simulink model "IEEE_34_node_2024a"  (R2024a)
settings: Solver=FixedStepDiscrete  StartTime=0.0  StopTime=0.001  RelTol=1e-3
InitFcn:
  | power_34NodeTestFeeder_init;
  | power_34NodeTestFeeder_loads_init;
  | %=============================
  | Frequency = 60;
  | 
  | Vnom_ph_ph = 24900;
  | Vnom = Vnom_ph_ph/sqrt(3);
  | 
  | Vnom1_ph_ph = 4160;
  | Vnom1 = Vnom1_ph_ph/sqrt(3);
  | 
  | Apparent_power_gen1 = 2500e3;
  | Degrees_ph_ph_gen1 = 0;
values from neighbouring files (symbols this model uses):
  C_300 = B_300/mi2km*ms2F    [power_34NodeTestFeeder_init.m]
  C_301 = B_301/mi2km*ms2F    [power_34NodeTestFeeder_init.m]
  C_302 = B_302/mi2km*ms2F    [power_34NodeTestFeeder_init.m]
  C_303 = B_303/mi2km*ms2F    [power_34NodeTestFeeder_init.m]
  C_304 = B_304/mi2km*ms2F    [power_34NodeTestFeeder_init.m]
  ft2km = 0.0003048    [power_34NodeTestFeeder_init.m]
  L_300 = X_300/mi2km/2/pi/60    [power_34NodeTestFeeder_init.m]
  L_301 = X_301/mi2km/2/pi/60    [power_34NodeTestFeeder_init.m]
  L_302 = X_302/mi2km/2/pi/60    [power_34NodeTestFeeder_init.m]
  L_303 = X_303/mi2km/2/pi/60    [power_34NodeTestFeeder_init.m]
  L_304 = X_304/mi2km/2/pi/60    [power_34NodeTestFeeder_init.m]
  Pa_802_806 = [1e-6 30 25]*1e3    [power_34NodeTestFeeder_loads_init.m]
  Pa_808_810 = 16e3    (= 16000)    [power_34NodeTestFeeder_loads_init.m]
  Pa_816_824 = [1e-6 5 1e-6]*1e3    [power_34NodeTestFeeder_loads_init.m]
  Pa_818_820 = 34e3    (= 34000)    [power_34NodeTestFeeder_loads_init.m]
  Pa_820_822 = 135e3    (= 135000)    [power_34NodeTestFeeder_loads_init.m]
  Pa_824_826 = 40e3    (= 40000)    [power_34NodeTestFeeder_loads_init.m]
  Pa_824_828 = [1e-6 1e-6 4]*1e3    [power_34NodeTestFeeder_loads_init.m]
  Pa_828_830 = [7 1e-6 1e-6]*1e3    [power_34NodeTestFeeder_loads_init.m]
  Pa_832_858 = [7 2 6]*1e3    [power_34NodeTestFeeder_loads_init.m]
  Pa_834_860 = [16 20 110]*1e3    [power_34NodeTestFeeder_loads_init.m]
  Pa_836_840 = [18 22 1e-6]*1e3    [power_34NodeTestFeeder_loads_init.m]
  Pa_842_844 = [9 1e-6 1e-6]*1e3    [power_34NodeTestFeeder_loads_init.m]
  Pa_844_846 = [1e-6 25 20]*1e3    [power_34NodeTestFeeder_loads_init.m]
  Pa_846_848 = [1e-6 23 1e-6]*1e3    [power_34NodeTestFeeder_loads_init.m]
  Pa_854_856 = 4e3    (= 4000)    [power_34NodeTestFeeder_loads_init.m]
  Pa_858_834 = [4 15 13]*1e3    [power_34NodeTestFeeder_loads_init.m]
  Pa_858_864 = 2e3    (= 2000)    [power_34NodeTestFeeder_loads_init.m]
  Pa_860_836 = [30 10 42]*1e3    [power_34NodeTestFeeder_loads_init.m]
  Pa_862_838 = 28e3    (= 28000)    [power_34NodeTestFeeder_loads_init.m]
  Pp_802_806 = [1e-6 15 14]*1e3    [power_34NodeTestFeeder_loads_init.m]
  Pp_808_810 = 8e3    (= 8000)    [power_34NodeTestFeeder_loads_init.m]
  Pp_816_824 = [1e-6 2 1e-6]*1e3    [power_34NodeTestFeeder_loads_init.m]
  Pp_818_820 = 17e3    (= 17000)    [power_34NodeTestFeeder_loads_init.m]
  Pp_820_822 = 70e3    (= 70000)    [power_34NodeTestFeeder_loads_init.m]
  Pp_824_826 = 20e3    (= 20000)    [power_34NodeTestFeeder_loads_init.m]
  Pp_824_828 = [1e-6 1e-6 2]*1e3    [power_34NodeTestFeeder_loads_init.m]
  Pp_828_830 = [3 1e-6 1e-6]*1e3    [power_34NodeTestFeeder_loads_init.m]
  Pp_832_858 = [3 1 3]*1e3    [power_34NodeTestFeeder_loads_init.m]
  Pp_834_860 = [8 10 55]*1e3    [power_34NodeTestFeeder_loads_init.m]
  Pp_836_840 = [9 11 1e-6]*1e3    [power_34NodeTestFeeder_loads_init.m]
  Pp_842_844 = [5 1e-6 1e-6]*1e3    [power_34NodeTestFeeder_loads_init.m]
  Pp_844_846 = [1e-6 12 11]*1e3    [power_34NodeTestFeeder_loads_init.m]
  Pp_846_848 = [1e-6 11 1e-6]*1e3    [power_34NodeTestFeeder_loads_init.m]
  Pp_854_856 = 2e3    (= 2000)    [power_34NodeTestFeeder_loads_init.m]
  Pp_858_834 = [2 8 7]*1e3    [power_34NodeTestFeeder_loads_init.m]
  Pp_858_864 = 1e3    (= 1000)    [power_34NodeTestFeeder_loads_init.m]
  Pp_860_836 = [15 6 22]*1e3    [power_34NodeTestFeeder_loads_init.m]
  Pp_862_838 = 14e3    (= 14000)    [power_34NodeTestFeeder_loads_init.m]
  R_300 = R_300/mi2km    [power_34NodeTestFeeder_init.m]
  R_301 = R_301/mi2km    [power_34NodeTestFeeder_init.m]
  R_302 = R_302/mi2km    [power_34NodeTestFeeder_init.m]
  R_303 = R_303/mi2km    [power_34NodeTestFeeder_init.m]
  R_304 = R_304/mi2km    [power_34NodeTestFeeder_init.m]
  V_802_806 = [1.047 1.048 1.048]    [power_34NodeTestFeeder_loads_init.m]
  V_808_810 = 1.014    [power_34NodeTestFeeder_loads_init.m]
  V_816_824 = [1.017 1.025 1.02]*sqrt(3)    [power_34NodeTestFeeder_loads_init.m]
  V_818_820 = 1.016    [power_34NodeTestFeeder_loads_init.m]
  V_820_822 = 0.993    [power_34NodeTestFeeder_loads_init.m]
  V_824_826 = 1.008    [power_34NodeTestFeeder_loads_init.m]
  V_824_828 = [1.008 1.016 1.012]    [power_34NodeTestFeeder_loads_init.m]
  V_828_830 = [1.007 1.015 1.011]    [power_34NodeTestFeeder_loads_init.m]
  V_830 = [0.989 0.998 0.994]*sqrt(3)    [power_34NodeTestFeeder_loads_init.m]
  V_832_858 = [1.036 1.035 1.036]*sqrt(3)    [power_34NodeTestFeeder_loads_init.m]
  V_834_860 = [1.031 1.029 1.031]*sqrt(3)    [power_34NodeTestFeeder_loads_init.m]
  V_836_840 = [1.03 1.029 1.031]*sqrt(3)    [power_34NodeTestFeeder_loads_init.m]
  V_840 = [1.03 1.029 1.031]    [power_34NodeTestFeeder_loads_init.m]
  V_842_844 = [1.031 1.029 1.031]    [power_34NodeTestFeeder_loads_init.m]
  V_844 = [1.031 1.029 1.031]    [power_34NodeTestFeeder_loads_init.m]
  V_844_846 = [1.031 1.029 1.031]    [power_34NodeTestFeeder_loads_init.m]
  V_846_848 = [1.031 1.029 1.031]    [power_34NodeTestFeeder_loads_init.m]
  V_848 = [1.031 1.029 1.031]*sqrt(3)    [power_34NodeTestFeeder_loads_init.m]
  V_854_856 = 0.998    [power_34NodeTestFeeder_loads_init.m]
  V_858_834 = [1.034 1.032 1.034]*sqrt(3)    [power_34NodeTestFeeder_loads_init.m]
  V_860 = [1.03 1.029 1.031]    [power_34NodeTestFeeder_loads_init.m]
  V_860_836 = [1.03 1.029 1.031]*sqrt(3)    [power_34NodeTestFeeder_loads_init.m]
  V_862_838 = 1.029    [power_34NodeTestFeeder_loads_init.m]
  V_890 = [0.917 0.924 0.918]*sqrt(3)    [power_34NodeTestFeeder_loads_init.m]
undefined symbols (not defined in the file or in its folder): Angle, D1, Degrees_ph, Delta, Magnitude, ph, ph_gen1, Vnom_ph
libraries: spsLoadFlowBusLib x36, spsDistributedParametersLineLib x32, spsThreePhaseSeriesRLCLoadLib x32, spsThreePhaseVIMeasurementLib x17, spsSeriesRLCLoadLib x14, spsGroundLib x14, spsThreePhaseSourceLib x1, spsThreePhaseTransformerTwoWindingsLib x1, sps_lib x1
(part 1 of 2)
settings Model: Creator=liyifu  LastModifiedBy=GW SmartGrid Lab
settings SolverCC: AlgebraicLoopSolver=TrustRegion
settings DataIOCC: ReturnWorkspaceOutputs=off
settings DebuggingCC: UnconnectedInputMsg=warning  UnconnectedOutputMsg=warning  UnconnectedLineMsg=warning  ArithmeticOperatorsInVariantConditions=warning
settings ModelReferenceCC: EnableRefExpFcnMdlSchedulingChecks=on
settings SFSimCC: SimUseLocalCustomCode=off  CustomCodeUndefinedFunction=UseInterfaceOnly
settings RTWCC: RTWUseLocalCustomCode=off  GPUComputeCapability=3.5

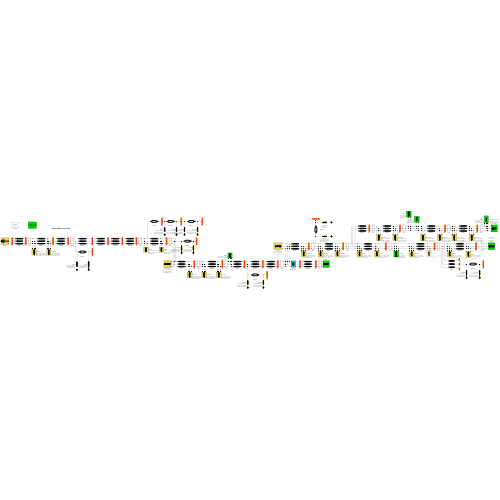
[thumbnail: Simulink's stored preview of the top-level diagram]
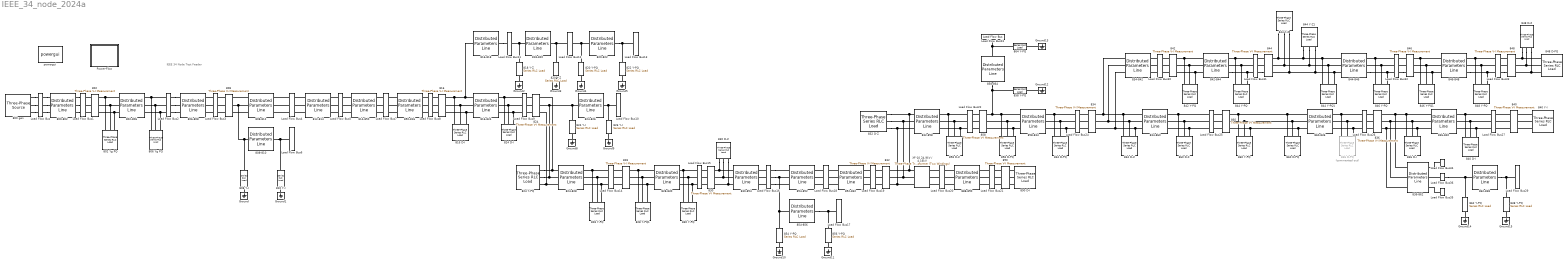
== system IEEE_34_node_2024a ==
  note: IEEE 34 Node Test Feeder
  800 gen : spsThreePhaseSourceLib/Three-Phase Source  InternalConnection=Yg  VoltagePhases=on  Voltage=Vnom_ph-ph  PhaseAngle=Degrees_ph-ph_gen1  Voltage_phases=[1.05 1.05 1.05]*Vnom  PhaseAngles_phases=[0 -120 +120]  Frequency=Frequency  NonIdealSource=off  SpecifyImpedance=on  Resistance=0.8929  Inductance=16.58e-3  ShortCircuitLevel=Apparent_power_gen1  BaseVoltage=Vnom_ph_ph  XRratio=8  BusType=swing  Pref=10e3  Qref=0  Prefabc=[10e3, 10e3, 10e3]  Qrefabc=[0, 0, 0]  Qmin=-inf  Qmax=inf
  800-802 : spsDistributedParametersLineLib/Distributed Parameters Line  Phases=3  Length=2580*ft2km  Frequency=Frequency  Resistance=R_300  Inductance=L_300  Capacitance=C_300  x1=[-3.47e-06 1164.97070083718 7669.53294066039 0;-3.46e-06 1164.9689933214 7669.62711521654 0;-3.45e-06 1164.96728578908 7669.72128966369 0;-3.44e-06 1164.96557824019 7669.81546400184 0;-3.43e-06 1164.96387067475 7669.90963823098 0;-3.42e-06 1164.96216309275 7670.00381235112 0;-3.41e-06 1164.96045549419 7670.09798636224 0;-3.4e-06 1164.95874787908 7670.19216026436 0;-3.39e-06 1164.95704024741 7670.28633405747 0;-3.38e-06 1164.95533259918 7670.38050774156 0;-3.37e-06 1164.9536249344 7670.47468131665 0;-3.36e-06 1164.95191725306 7670.56885478272 0;-3.35e-06 1164.95020955516 7670.66302813977 0;-3.34e-06 1164.94850184071 7670.7572013878 0;-3.33e-06 1164.9467941097 7670.85137452682 0;-3.32e-06 1164.94508636213 7670.94554755682 0;-3.31e-06 1164.94337859801 7671.03972047779 0;-3.3e-06 1164.94167081733 7671.13389328974 0;-3.29e-06 1164.9399630201 7671.22806599267 0;-3.28e-06 1164.93825520631 7671.32223858658 0;-3.27e-06 1164.93654737596 7671.41641107145 0;-3.26e-06 1164.93483952905 7671.5105834473 0;-3.25e-06 1164.93313166559 7671.60475571412 0;-3.24e-06 1164.93142378558 7671.69892787191 0;-3.23e-06 1164.929715889 7671.79309992067 0;-3.22e-06 1164.92800797587 7671.88727186039 0;-3.21e-06 1164.92630004619 7671.98144369108 0;-3.2e-06 1164.92459209995 7672.07561541273 0;-3.19e-06 1164.92288413715 7672.16978702535 0;-3.18e-06 1164.9211761578 7672.26395852893 0;-3.17e-06 1164.91946816189 7672.35812992346 0;-3.16e-06 1164.91776014942 7672.45230120896 0;-3.15e-06 1164.9160521204 7672.54647238541 0;-3.14e-06 1164.91434407482 7672.64064345282 0;-3.13e-06 1164.91263601268 7672.73481441119 0;-3.12e-06 1164.91092793399 7672.8289852605 0;-3.11e-06 1164.90921983875 7672.92315600077 0;-3.1e-06 1164.90751172694 7673.01732663199 0;-3.09e-06 1164.90580359859 7673.11149715416 0;-3.08e-06 1164.90409545367 7673.20566756728 0;-3.07e-06 1164.9023872922 7673.29983787134 0;-3.06e-06 1164.90067911418 7673.39400806635 0;-3.05e-06 1164.89897091959 7673.48817815231 0;-3.04e-06 1164.89726270846 7673.5823481292 0;-3.03e-06 1164.89555448076 7673.67651799704 0;-3.02e-06 1164.89384623651 7673.77068775581 0;-3.01e-06 1164.89213797571 7673.86485740553 0;-3e-06 1164.89042969835 7673.95902694618 0;-2.99e-06 1164.88872140443 7674.05319637777 0;-2.98e-06 1164.88701309396 7674.1473657003 0;-2.97e-06 1164.88530476693 7674.24153491375 0;-2.96e-06 1164.88359642335 7674.33570401814 0;-2.95e-06 1164.88188806321 7674.42987301346 0;-2.94e-06 1164.88017968652 7674.52404189971 0;-2.93e-06 1164.87847129326 7674.61821067688 0;-2.92e-06 1164.87676288346 7674.71237934499 0;-2.91e-06 1164.8750544571 7674.80654790401 0;-2.9e-06 1164.87334601418 7674.90071635397 24835.4217460891;-2.89e-06 1164.87163755471 7674.99488469484 24835.3926245131;-2.88e-06 1164.86992907868 7675.08905292664 24835.3635025841;-2.87e-06 1164.8682205861 7675.18322104935 24835.3343803022;-2.86e-06 1164.86651207696 7675.27738906298 24835.3052576673;-2.85e-06 1164.86480355127 7675.37155696754 24835.2761346794;-2.84e-06 1164.86309500902 7675.465724763 24835.2470113386;-2.83e-06 1164.86138645022 7675.55989244939 24835.2178876448;-2.82e-06 1164.85967787486 7675.65406002668 24835.188763598;-2.81e-06 1164.85796928294 7675.74822749489 24835.1596391983;-2.8e-06 1164.85626067447 7675.842394854 24835.1305144456;-2.79e-06 1164.85455204945 7675.93656210403 24835.10138934;-2.78e-06 1164.85284340787 7676.03072924496 24835.0722638813;-2.77e-06 1164.85113474973 7676.1248962768 24835.0431380698;-2.76e-06 1164.84942607504 7676.21906319955 24835.0140119052;-2.75e-06 1164.84771738379 7676.3132300132 24834.9848853877;-2.74e-06 1164.84600867599 7676.40739671775 24834.9557585173;-2.73e-06 1164.84429995164 7676.5015633132 24834.9266312939;-2.72e-06 1164.84259121073 7676.59572979956 24834.8975037175;-2.71e-06 1164.84088245326 7676.68989617681 24834.8683757881;-2.7e-06 1164.83917367924 7676.78406244496 24834.8392475058;-2.69e-06 1164.83746488866 7676.878228604 24834.8101188706;-2.68e-06 1164.83575608153 7676.97239465394 24834.7809898824;-2.67e-06 ...(+16035 chars)]  x2=[-3.47e-06 1168.50780914208 7624.72535894941 0;-3.46e-06 1168.50605789348 7624.81945720973 0;-3.45e-06 1168.50430662827 7624.91355536169 0;-3.44e-06 1168.50255534646 7625.00765340529 0;-3.43e-06 1168.50080404804 7625.10175134052 0;-3.42e-06 1168.49905273301 7625.19584916737 0;-3.41e-06 1168.49730140137 7625.28994688586 0;-3.4e-06 1168.49555005313 7625.38404449597 0;-3.39e-06 1168.49379868828 7625.47814199771 0;-3.38e-06 1168.49204730682 7625.57223939107 0;-3.37e-06 1168.49029590876 7625.66633667606 0;-3.36e-06 1168.48854449409 7625.76043385267 0;-3.35e-06 1168.48679306282 7625.8545309209 0;-3.34e-06 1168.48504161493 7625.94862788076 0;-3.33e-06 1168.48329015044 7626.04272473222 0;-3.32e-06 1168.48153866934 7626.13682147531 0;-3.31e-06 1168.47978717164 7626.23091811001 0;-3.3e-06 1168.47803565733 7626.32501463633 0;-3.29e-06 1168.47628412641 7626.41911105426 0;-3.28e-06 1168.47453257889 7626.5132073638 0;-3.27e-06 1168.47278101476 7626.60730356495 0;-3.26e-06 1168.47102943402 7626.70139965771 0;-3.25e-06 1168.46927783668 7626.79549564207 0;-3.24e-06 1168.46752622273 7626.88959151805 0;-3.23e-06 1168.46577459217 7626.98368728562 0;-3.22e-06 1168.46402294501 7627.07778294481 0;-3.21e-06 1168.46227128124 7627.17187849559 0;-3.2e-06 1168.46051960086 7627.26597393798 0;-3.19e-06 1168.45876790388 7627.36006927196 0;-3.18e-06 1168.45701619029 7627.45416449754 0;-3.17e-06 1168.45526446009 7627.54825961472 0;-3.16e-06 1168.45351271329 7627.6423546235 0;-3.15e-06 1168.45176094988 7627.73644952387 0;-3.14e-06 1168.45000916987 7627.83054431583 0;-3.13e-06 1168.44825737325 7627.92463899938 0;-3.12e-06 1168.44650556002 7628.01873357452 0;-3.11e-06 1168.44475373019 7628.11282804126 0;-3.1e-06 1168.44300188375 7628.20692239958 0;-3.09e-06 1168.4412500207 7628.30101664948 0;-3.08e-06 1168.43949814105 7628.39511079098 0;-3.07e-06 1168.43774624479 7628.48920482405 0;-3.06e-06 1168.43599433193 7628.58329874871 0;-3.05e-06 1168.43424240246 7628.67739256494 0;-3.04e-06 1168.43249045638 7628.77148627276 0;-3.03e-06 1168.4307384937 7628.86557987216 0;-3.02e-06 1168.42898651441 7628.95967336313 0;-3.01e-06 1168.42723451852 7629.05376674567 0;-3e-06 1168.42548250602 7629.1478600198 0;-2.99e-06 1168.42373047691 7629.24195318549 0;-2.98e-06 1168.4219784312 7629.33604624276 0;-2.97e-06 1168.42022636888 7629.43013919159 0;-2.96e-06 1168.41847428996 7629.524232032 0;-2.95e-06 1168.41672219443 7629.61832476397 0;-2.94e-06 1168.4149700823 7629.71241738751 0;-2.93e-06 1168.41321795355 7629.80650990261 0;-2.92e-06 1168.41146580821 7629.90060230928 0;-2.91e-06 1168.40971364626 7629.9946946075 0;-2.9e-06 1168.4079614677 7630.08878679729 24823.450895793;-2.89e-06 1168.40620927253 7630.18287887864 24823.4219609793;-2.88e-06 1168.40445706076 7630.27697085155 24823.3930258128;-2.87e-06 1168.40270483239 7630.37106271601 24823.3640902935;-2.86e-06 1168.40095258741 7630.46515447203 24823.3351544214;-2.85e-06 1168.39920032582 7630.5592461196 24823.3062181966;-2.84e-06 1168.39744804763 7630.65333765873 24823.2772816189;-2.83e-06 1168.39569575283 7630.74742908941 24823.2483446885;-2.82e-06 1168.39394344143 7630.84152041163 24823.2194074052;-2.81e-06 1168.39219111342 7630.93561162541 24823.1904697692;-2.8e-06 1168.39043876881 7631.02970273073 24823.1615317803;-2.79e-06 1168.38868640759 7631.1237937276 24823.1325934387;-2.78e-06 1168.38693402976 7631.21788461602 24823.1036547443;-2.77e-06 1168.38518163533 7631.31197539597 24823.0747156971;-2.76e-06 1168.3834292243 7631.40606606747 24823.0457762971;-2.75e-06 1168.38167679665 7631.50015663051 24823.0168365443;-2.74e-06 1168.37992435241 7631.59424708509 24822.9878964387;-2.73e-06 1168.37817189156 7631.68833743121 24822.9589559803;-2.72e-06 1168.3764194141 7631.78242766887 24822.9300151692;-2.71e-06 1168.37466692004 7631.87651779805 24822.9010740052;-2.7e-06 1168.37291440937 7631.97060781878 24822.8721324885;-2.69e-06 1168.3711618821 7632.06469773104 24822.8431906189;-2.68e-06 1168.36940933822 7632.15878753482 24822.8142483966 ...(+16047 chars)]  x3=[-3.47e-06 9.92857968224924 -15.9400911726028 0;-3.46e-06 9.9285662285998 -15.9400481932344 0;-3.45e-06 9.92855277480924 -15.9400052136394 0;-3.44e-06 9.92853932087758 -15.9399622338179 0;-3.43e-06 9.92852586680481 -15.9399192537698 0;-3.42e-06 9.92851241259094 -15.9398762734952 0;-3.41e-06 9.92849895823595 -15.939833292994 0;-3.4e-06 9.92848550373987 -15.9397903122663 0;-3.39e-06 9.92847204910267 -15.9397473313121 0;-3.38e-06 9.92845859432437 -15.9397043501313 0;-3.37e-06 9.92844513940497 -15.939661368724 0;-3.36e-06 9.92843168434446 -15.9396183870901 0;-3.35e-06 9.92841822914284 -15.9395754052297 0;-3.34e-06 9.92840477380012 -15.9395324231428 0;-3.33e-06 9.9283913183163 -15.9394894408293 0;-3.32e-06 9.92837786269137 -15.9394464582893 0;-3.31e-06 9.92836440692534 -15.9394034755228 0;-3.3e-06 9.9283509510182 -15.9393604925297 0;-3.29e-06 9.92833749496996 -15.9393175093101 0;-3.28e-06 9.92832403878061 -15.9392745258639 0;-3.27e-06 9.92831058245016 -15.9392315421913 0;-3.26e-06 9.92829712597861 -15.9391885582921 0;-3.25e-06 9.92828366936595 -15.9391455741663 0;-3.24e-06 9.92827021261219 -15.9391025898141 0;-3.23e-06 9.92825675571733 -15.9390596052353 0;-3.22e-06 9.92824329868137 -15.9390166204299 0;-3.21e-06 9.9282298415043 -15.9389736353981 0;-3.2e-06 9.92821638418613 -15.9389306501397 0;-3.19e-06 9.92820292672686 -15.9388876646548 0;-3.18e-06 9.92818946912648 -15.9388446789433 0;-3.17e-06 9.92817601138501 -15.9388016930054 0;-3.16e-06 9.92816255350243 -15.9387587068409 0;-3.15e-06 9.92814909547876 -15.9387157204499 0;-3.14e-06 9.92813563731398 -15.9386727338323 0;-3.13e-06 9.9281221790081 -15.9386297469883 0;-3.12e-06 9.92810872056111 -15.9385867599177 0;-3.11e-06 9.92809526197303 -15.9385437726206 0;-3.1e-06 9.92808180324385 -15.9385007850969 0;-3.09e-06 9.92806834437357 -15.9384577973468 0;-3.08e-06 9.92805488536219 -15.9384148093701 0;-3.07e-06 9.92804142620971 -15.9383718211669 0;-3.06e-06 9.92802796691612 -15.9383288327372 0;-3.05e-06 9.92801450748144 -15.9382858440809 0;-3.04e-06 9.92800104790566 -15.9382428551982 0;-3.03e-06 9.92798758818878 -15.9381998660889 0;-3.02e-06 9.92797412833081 -15.9381568767531 0;-3.01e-06 9.92796066833173 -15.9381138871908 0;-3e-06 9.92794720819155 -15.938070897402 0;-2.99e-06 9.92793374791028 -15.9380279073867 0;-2.98e-06 9.92792028748791 -15.9379849171448 0;-2.97e-06 9.92790682692444 -15.9379419266764 0;-2.96e-06 9.92789336621987 -15.9378989359816 0;-2.95e-06 9.92787990537421 -15.9378559450602 0;-2.94e-06 9.92786644438745 -15.9378129539123 0;-2.93e-06 9.92785298325959 -15.9377699625378 0;-2.92e-06 9.92783952199063 -15.9377269709369 0;-2.91e-06 9.92782606058058 -15.9376839791095 0;-2.9e-06 9.92781259902943 -15.9376409870555 3.85219105251387;-2.89e-06 9.92779913733718 -15.937597994775 3.85228642493883;-2.88e-06 9.92778567550384 -15.9375550022681 3.85238179730904;-2.87e-06 9.9277722135294 -15.9375120095346 3.8524771696245;-2.86e-06 9.92775875141387 -15.9374690165746 3.8525725418852;-2.85e-06 9.92774528915724 -15.9374260233881 3.85266791409115;-2.84e-06 9.92773182675952 -15.9373830299751 3.85276328624235;-2.83e-06 9.9277183642207 -15.9373400363356 3.85285865833879;-2.82e-06 9.92770490154078 -15.9372970424696 3.85295403038047;-2.81e-06 9.92769143871977 -15.9372540483771 3.85304940236739;-2.8e-06 9.92767797575767 -15.9372110540581 3.85314477429956;-2.79e-06 9.92766451265447 -15.9371680595126 3.85324014617696;-2.78e-06 9.92765104941018 -15.9371250647405 3.85333551799959;-2.77e-06 9.92763758602479 -15.937082069742 3.85343088976747;-2.76e-06 9.92762412249831 -15.937039074517 3.85352626148057;-2.75e-06 9.92761065883074 -15.9369960790655 3.85362163313892;-2.74e-06 9.92759719502207 -15.9369530833874 3.85371700474249;-2.73e-06 9.92758373107231 -15.9369100874829 3.85381237629129;-2.72e-06 9.92757026698145 -15.9368670913519 3.85390774778532;-2.71e-06 9.9275568027495 -15.9368240949943 3.85400311922458;-2.7e-06 9.92754333837646 -15.9367810984103 3.85409849060906;-2.69e-06 9.92752987386233 ...(+16395 chars)]  x4=[-3.47e-06 -7.10509597593038 61.6147676127846 0;-3.46e-06 -7.10508669932798 61.6152880096286 0;-3.45e-06 -7.10507742262459 61.615808405597 0;-3.44e-06 -7.10506814582023 61.6163288006896 0;-3.43e-06 -7.10505886891489 61.6168491949066 0;-3.42e-06 -7.10504959190858 61.6173695882478 0;-3.41e-06 -7.10504031480128 61.6178899807134 0;-3.4e-06 -7.105031037593 61.6184103723031 0;-3.39e-06 -7.10502176028375 61.6189307630172 0;-3.38e-06 -7.10501248287352 61.6194511528555 0;-3.37e-06 -7.10500320536231 61.6199715418181 0;-3.36e-06 -7.10499392775012 61.6204919299049 0;-3.35e-06 -7.10498465003696 61.6210123171159 0;-3.34e-06 -7.10497537222281 61.6215327034512 0;-3.33e-06 -7.10496609430769 61.6220530889107 0;-3.32e-06 -7.10495681629159 61.6225734734944 0;-3.31e-06 -7.10494753817452 61.6230938572023 0;-3.3e-06 -7.10493825995647 61.6236142400344 0;-3.29e-06 -7.10492898163744 61.6241346219907 0;-3.28e-06 -7.10491970321743 61.6246550030712 0;-3.27e-06 -7.10491042469645 61.6251753832758 0;-3.26e-06 -7.10490114607449 61.6256957626047 0;-3.25e-06 -7.10489186735155 61.6262161410576 0;-3.24e-06 -7.10488258852764 61.6267365186348 0;-3.23e-06 -7.10487330960275 61.6272568953361 0;-3.22e-06 -7.10486403057688 61.6277772711615 0;-3.21e-06 -7.10485475145004 61.6282976461111 0;-3.2e-06 -7.10484547222223 61.6288180201847 0;-3.19e-06 -7.10483619289343 61.6293383933826 0;-3.18e-06 -7.10482691346367 61.6298587657045 0;-3.17e-06 -7.10481763393292 61.6303791371505 0;-3.16e-06 -7.1048083543012 61.6308995077206 0;-3.15e-06 -7.10479907456851 61.6314198774148 0;-3.14e-06 -7.10478979473484 61.6319402462331 0;-3.13e-06 -7.1047805148002 61.6324606141754 0;-3.12e-06 -7.10477123476458 61.6329809812418 0;-3.11e-06 -7.10476195462798 61.6335013474323 0;-3.1e-06 -7.10475267439042 61.6340217127468 0;-3.09e-06 -7.10474339405187 61.6345420771854 0;-3.08e-06 -7.10473411361236 61.635062440748 0;-3.07e-06 -7.10472483307187 61.6355828034346 0;-3.06e-06 -7.1047155524304 61.6361031652453 0;-3.05e-06 -7.10470627168796 61.6366235261799 0;-3.04e-06 -7.10469699084455 61.6371438862386 0;-3.03e-06 -7.10468770990017 61.6376642454212 0;-3.02e-06 -7.10467842885481 61.6381846037279 0;-3.01e-06 -7.10466914770848 61.6387049611586 0;-3e-06 -7.10465986646117 61.6392253177132 0;-2.99e-06 -7.10465058511289 61.6397456733918 0;-2.98e-06 -7.10464130366364 61.6402660281943 0;-2.97e-06 -7.10463202211341 61.6407863821208 0;-2.96e-06 -7.10462274046222 61.6413067351713 0;-2.95e-06 -7.10461345871005 61.6418270873457 0;-2.94e-06 -7.10460417685691 61.642347438644 0;-2.93e-06 -7.10459489490279 61.6428677890662 0;-2.92e-06 -7.1045856128477 61.6433881386124 0;-2.91e-06 -7.10457633069165 61.6439084872825 0;-2.9e-06 -7.10456704843461 61.6444288350765 119.785457609531;-2.89e-06 -7.10455776607661 61.6449491819943 119.78521797053;-2.88e-06 -7.10454848361764 61.6454695280361 119.784978329827;-2.87e-06 -7.10453920105769 61.6459898732017 119.784738687421;-2.86e-06 -7.10452991839678 61.6465102174912 119.784499043313;-2.85e-06 -7.10452063563489 61.6470305609046 119.784259397502;-2.84e-06 -7.10451135277203 61.6475509034419 119.784019749989;-2.83e-06 -7.1045020698082 61.648071245103 119.783780100774;-2.82e-06 -7.1044927867434 61.6485915858879 119.783540449856;-2.81e-06 -7.10448350357763 61.6491119257967 119.783300797236;-2.8e-06 -7.10447422031089 61.6496322648293 119.783061142913;-2.79e-06 -7.10446493694317 61.6501526029857 119.782821486888;-2.78e-06 -7.10445565347449 61.6506729402659 119.782581829161;-2.77e-06 -7.10444636990484 61.6511932766699 119.782342169731;-2.76e-06 -7.10443708623422 61.6517136121978 119.782102508599;-2.75e-06 -7.10442780246262 61.6522339468494 119.781862845764;-2.74e-06 -7.10441851859006 61.6527542806248 119.781623181228;-2.73e-06 -7.10440923461653 61.653274613524 119.781383514988;-2.72e-06 -7.10439995054203 61.653794945547 119.781143847047;-2.71e-06 -7.10439066636656 61.6543152766937 119.780904177403;-2.7e-06 -7.10438138209012 61.6548356069642 119.780664506057;-2.69e-06 -7.10437209771271 ...(+16405 chars)]  x5=3.46223763231864e-06  V1=ones(3,3)  V2=ones(3,3)  I1=ones(3,3)  I2=ones(3,3)  nHarmo=1  Measurements=None  Decoupling=off  VsMag0=0  VrMag0=0  VsAngle0=0  VrAngle0=0  IsMag0=0  IrMag0=0  IsAngle0=0  IrAngle0=0
      settings: LibrarySourceBlock=sps_lib/Power Grid Elements/Distributed Parameters Line
  802 : spsThreePhaseVIMeasurementLib/Three-Phase V-I Measurement  VoltageMeasurement=phase-to-ground  SetLabelV=on  LabelV=Vabc_802  Vpu=on  VpuLL=off  CurrentMeasurement=yes  SetLabelI=on  LabelI=Iabc_802  Ipu=on  Pbase=100e6  Vbase=Vnom_ph_ph  OutputType=Magnitude-Angle  PhasorSimulation=off  PSBequivalent=0
  802 Yg PQ : spsThreePhaseSeriesRLCLoadLib/Three-Phase Series RLC Load  LConnTagsString=A|B|C  Configuration=Y (grounded)  NominalVoltage=[  Vabc=V_802_806*Vnom  Vabcp=[1e3 1e3 1e3]  NominalFrequency=Frequency  UnbalancedPower=on  ActivePower=10e3  InductivePower=100  CapacitivePower=100  Pabc=Pa_802_806/2  QLabc=Pp_802_806/2  QCabc=Pn_802_806  Pabcp=[10e3 9e3 11e3]  QLabcp=[100 90 110]  QCabcp=[100 90 110]  Measurements=None  LoadType=constant PQ
      settings: AttributesFormatString=|
      | P= %<Pabc> W
      | Ql=%<QLabc> var
      | Qc=%<QCabc> var
  802-806 : spsDistributedParametersLineLib/Distributed Parameters Line  Phases=3  Length=1730*ft2km  Frequency=Frequency  Resistance=R_300  Inductance=L_300  Capacitance=C_300  x1=[-2.33e-06 1168.30805984145 0 0;-2.32e-06 1168.3063066998 0 0;-2.31e-06 1168.30455354155 0 0;-2.3e-06 1168.3028003667 0 0;-2.29e-06 1168.30104717524 0 0;-2.28e-06 1168.29929396717 0 0;-2.27e-06 1168.29754074251 0 0;-2.26e-06 1168.29578750123 0 0;-2.25e-06 1168.29403424336 0 0;-2.24e-06 1168.29228096888 0 0;-2.23e-06 1168.29052767779 0 0;-2.22e-06 1168.28877437011 0 0;-2.21e-06 1168.28702104581 0 0;-2.2e-06 1168.28526770492 0 0;-2.19e-06 1168.28351434742 0 0;-2.18e-06 1168.28176097331 0 0;-2.17e-06 1168.28000758261 0 0;-2.16e-06 1168.2782541753 0 0;-2.15e-06 1168.27650075138 0 0;-2.14e-06 1168.27474731086 0 0;-2.13e-06 1168.27299385374 0 0;-2.12e-06 1168.27124038001 0 0;-2.11e-06 1168.26948688968 0 0;-2.1e-06 1168.26773338275 0 0;-2.09e-06 1168.26597985921 0 0;-2.08e-06 1168.26422631907 0 0;-2.07e-06 1168.26247276232 0 0;-2.06e-06 1168.26071918897 0 0;-2.05e-06 1168.25896559902 0 0;-2.04e-06 1168.25721199246 0 0;-2.03e-06 1168.25545836931 0 0;-2.02e-06 1168.25370472954 0 0;-2.01e-06 1168.25195107318 0 0;-2e-06 1168.25019740021 0 0;-1.99e-06 1168.24844371063 0 0;-1.98e-06 1168.24669000446 7638.74480417558 0;-1.97e-06 1168.24493628168 7638.83888627467 0;-1.96e-06 1168.24318254229 7638.9329682652 0;-1.95e-06 1168.2414287863 7639.02705014717 24820.7005133149;-1.94e-06 1168.23967501371 7639.12113192056 24820.6715449874;-1.93e-06 1168.23792122452 7639.21521358539 24820.6425763072;-1.92e-06 1168.23616741872 7639.30929514165 24820.6136072742;-1.91e-06 1168.23441359632 7639.40337658933 24820.5846378884;-1.9e-06 1168.23265975732 7639.49745792844 24820.5556681499;-1.89e-06 1168.23090590171 7639.59153915898 24820.5266980587;-1.88e-06 1168.2291520295 7639.68562028094 24820.4977276146;-1.87e-06 1168.22739814069 7639.77970129433 24820.4687568179;-1.86e-06 1168.22564423528 7639.87378219914 24820.4397856683;-1.85e-06 1168.22389031326 7639.96786299536 24820.4108141661;-1.84e-06 1168.22213637463 7640.06194368301 24820.381842311;-1.83e-06 1168.22038241941 7640.15602426207 24820.3528701032;-1.82e-06 1168.21862844758 7640.25010473255 24820.3238975427;-1.81e-06 1168.21687445915 7640.34418509445 24820.2949246294;-1.8e-06 1168.21512045412 7640.43826534776 24820.2659513633;-1.79e-06 1168.21336643248 7640.53234549248 24820.2369777445;-1.78e-06 1168.21161239424 7640.62642552861 24820.208003773;-1.77e-06 1168.2098583394 7640.72050545615 24820.1790294487;-1.76e-06 1168.20810426795 7640.8145852751 24820.1500547716;-1.75e-06 1168.2063501799 7640.90866498546 24820.1210797418;-1.74e-06 1168.20459607525 7641.00274458722 24820.0921043593;-1.73e-06 1168.202841954 7641.09682408039 24820.063128624;-1.72e-06 1168.20108781614 7641.19090346496 24820.0341525359;-1.71e-06 1168.19933366168 7641.28498274093 24820.0051760952;-1.7e-06 1168.19757949062 7641.37906190831 24819.9761993016;-1.69e-06 1168.19582530295 7641.47314096708 24819.9472221553;-1.68e-06 1168.19407109869 7641.56721991725 24819.9182446563;-1.67e-06 1168.19231687781 7641.66129875881 24819.8892668045;-1.66e-06 1168.19056264034 7641.75537749177 24819.8602886;-1.65e-06 1168.18880838627 7641.84945611612 24819.8313100427;-1.64e-06 1168.18705411559 7641.94353463187 24819.8023311327;-1.63e-06 1168.18529982831 7642.03761303901 24819.7733518699;-1.62e-06 1168.18354552442 7642.13169133753 24819.7443722544;-1.61e-06 1168.18179120394 7642.22576952745 24819.7153922862;-1.6e-06 1168.18003686685 7642.31984760875 24819.6864119652;-1.59e-06 1168.17828251316 7642.41392558144 24819.6574312914;-1.58e-06 1168.17652814287 7642.50800344551 24819.6284502649;-1.57e-06 1168.17477375597 7642.60208120096 24819.5994688857;-1.56e-06 1168.17301935247 7642.6961588478 24819.5704871538;-1.55e-06 1168.17126493237 7642.79023638601 24819.541505069;-1.54e-06 1168.16951049567 7642.88431381561 24819.5125226316;-1.53e-06 1168.16775604237 7642.97839113658 24819.4835398414;-1.52e-06 1168.16600157246 7643.07246834893 24819.4545566985;-1.51e-06 1168.16424708595 7643.16654545265 24819.4255732028;-1.5e-06 1168.16249258284 7643.26062244775 24819.3965893544 ...(+8899 chars)]  x2=[-2.33e-06 1170.22712194095 0 0;-2.32e-06 1170.22533716641 0 0;-2.31e-06 1170.22355237524 0 0;-2.3e-06 1170.22176756744 0 0;-2.29e-06 1170.219982743 0 0;-2.28e-06 1170.21819790193 0 0;-2.27e-06 1170.21641304423 0 0;-2.26e-06 1170.2146281699 0 0;-2.25e-06 1170.21284327894 0 0;-2.24e-06 1170.21105837135 0 0;-2.23e-06 1170.20927344712 0 0;-2.22e-06 1170.20748850627 0 0;-2.21e-06 1170.20570354878 0 0;-2.2e-06 1170.20391857466 0 0;-2.19e-06 1170.20213358391 0 0;-2.18e-06 1170.20034857653 0 0;-2.17e-06 1170.19856355252 0 0;-2.16e-06 1170.19677851188 0 0;-2.15e-06 1170.19499345461 0 0;-2.14e-06 1170.1932083807 0 0;-2.13e-06 1170.19142329017 0 0;-2.12e-06 1170.189638183 0 0;-2.11e-06 1170.1878530592 0 0;-2.1e-06 1170.18606791877 0 0;-2.09e-06 1170.18428276171 0 0;-2.08e-06 1170.18249758802 0 0;-2.07e-06 1170.1807123977 0 0;-2.06e-06 1170.17892719075 0 0;-2.05e-06 1170.17714196716 0 0;-2.04e-06 1170.17535672695 0 0;-2.03e-06 1170.1735714701 0 0;-2.02e-06 1170.17178619663 0 0;-2.01e-06 1170.17000090652 0 0;-2e-06 1170.16821559978 0 0;-1.99e-06 1170.16643027642 0 0;-1.98e-06 1170.16464493642 7609.01147985625 0;-1.97e-06 1170.16285957979 7609.10551159647 0;-1.96e-06 1170.16107420653 7609.19954322856 0;-1.95e-06 1170.15928881664 7609.2935747525 24813.4491375345;-1.94e-06 1170.15750341012 7609.3876061683 24813.4202937236;-1.93e-06 1170.15571798697 7609.48163747595 24813.3914495601;-1.92e-06 1170.15393254718 7609.57566867546 24813.362605044;-1.91e-06 1170.15214709077 7609.66969976681 24813.3337601752;-1.9e-06 1170.15036161773 7609.76373075001 24813.3049149537;-1.89e-06 1170.14857612805 7609.85776162507 24813.2760693796;-1.88e-06 1170.14679062175 7609.95179239197 24813.2472234528;-1.87e-06 1170.14500509882 7610.04582305071 24813.2183771734;-1.86e-06 1170.14321955925 7610.1398536013 24813.1895305414;-1.85e-06 1170.14143400306 7610.23388404373 24813.1606835566;-1.84e-06 1170.13964843023 7610.32791437801 24813.1318362193;-1.83e-06 1170.13786284077 7610.42194460412 24813.1029885292;-1.82e-06 1170.13607723469 7610.51597472207 24813.0741404866;-1.81e-06 1170.13429161197 7610.61000473187 24813.0452920913;-1.8e-06 1170.13250597263 7610.70403463349 24813.0164433433;-1.79e-06 1170.13072031665 7610.79806442695 24812.9875942427;-1.78e-06 1170.12893464404 7610.89209411225 24812.9587447894;-1.77e-06 1170.1271489548 7610.98612368938 24812.9298949835;-1.76e-06 1170.12536324894 7611.08015315834 24812.901044825;-1.75e-06 1170.12357752644 7611.17418251912 24812.8721943138;-1.74e-06 1170.12179178731 7611.26821177174 24812.8433434499;-1.73e-06 1170.12000603156 7611.36224091618 24812.8144922334;-1.72e-06 1170.11822025917 7611.45626995245 24812.7856406643;-1.71e-06 1170.11643447015 7611.55029888054 24812.7567887425;-1.7e-06 1170.1146486645 7611.64432770046 24812.7279364681;-1.69e-06 1170.11286284223 7611.7383564122 24812.699083841;-1.68e-06 1170.11107700332 7611.83238501576 24812.6702308613;-1.67e-06 1170.10929114778 7611.92641351113 24812.6413775289;-1.66e-06 1170.10750527562 7612.02044189833 24812.6125238439;-1.65e-06 1170.10571938682 7612.11447017734 24812.5836698063;-1.64e-06 1170.10393348139 7612.20849834816 24812.554815416;-1.63e-06 1170.10214755934 7612.3025264108 24812.525960673;-1.62e-06 1170.10036162065 7612.39655436525 24812.4971055775;-1.61e-06 1170.09857566533 7612.49058221151 24812.4682501293;-1.6e-06 1170.09678969339 7612.58460994959 24812.4393943284;-1.59e-06 1170.09500370481 7612.67863757946 24812.4105381749;-1.58e-06 1170.09321769961 7612.77266510115 24812.3816816688;-1.57e-06 1170.09143167777 7612.86669251464 24812.35282481;-1.56e-06 1170.08964563931 7612.96071981994 24812.3239675986;-1.55e-06 1170.08785958422 7613.05474701704 24812.2951100345;-1.54e-06 1170.08607351249 7613.14877410594 24812.2662521179;-1.53e-06 1170.08428742414 7613.24280108664 24812.2373938485;-1.52e-06 1170.08250131916 7613.33682795914 24812.2085352266;-1.51e-06 1170.08071519755 7613.43085472344 24812.179676252;-1.5e-06 1170.0789290593 7613.52488137953 24812.1508169247 ...(+8891 chars)]  x3=[-2.33e-06 10.1821600461527 0 0;-2.32e-06 10.1821434825898 0 0;-2.31e-06 10.1821269188822 0 0;-2.3e-06 10.1821103550299 0 0;-2.29e-06 10.1820937910329 0 0;-2.28e-06 10.1820772268912 0 0;-2.27e-06 10.1820606626047 0 0;-2.26e-06 10.1820440981736 0 0;-2.25e-06 10.1820275335977 0 0;-2.24e-06 10.1820109688771 0 0;-2.23e-06 10.1819944040118 0 0;-2.22e-06 10.1819778390018 0 0;-2.21e-06 10.1819612738471 0 0;-2.2e-06 10.1819447085477 0 0;-2.19e-06 10.1819281431036 0 0;-2.18e-06 10.1819115775148 0 0;-2.17e-06 10.1818950117813 0 0;-2.16e-06 10.181878445903 0 0;-2.15e-06 10.1818618798801 0 0;-2.14e-06 10.1818453137124 0 0;-2.13e-06 10.1818287474 0 0;-2.12e-06 10.181812180943 0 0;-2.11e-06 10.1817956143412 0 0;-2.1e-06 10.1817790475947 0 0;-2.09e-06 10.1817624807035 0 0;-2.08e-06 10.1817459136676 0 0;-2.07e-06 10.181729346487 0 0;-2.06e-06 10.1817127791617 0 0;-2.05e-06 10.1816962116917 0 0;-2.04e-06 10.181679644077 0 0;-2.03e-06 10.1816630763176 0 0;-2.02e-06 10.1816465084135 0 0;-2.01e-06 10.1816299403647 0 0;-2e-06 10.1816133721711 0 0;-1.99e-06 10.1815968038329 0 0;-1.98e-06 10.18158023535 -15.7066483743921 0;-1.97e-06 10.1815636667223 -15.7066026544002 0;-1.96e-06 10.18154709795 -15.7065569341851 0;-1.95e-06 10.181530529033 -15.7065112137468 5.36567394079704;-1.94e-06 10.1815139599712 -15.7064654930853 5.36577240394942;-1.93e-06 10.1814973907648 -15.7064197722006 5.36587086702554;-1.92e-06 10.1814808214136 -15.7063740510926 5.3659693300254;-1.91e-06 10.1814642519178 -15.7063283297614 5.36606779294899;-1.9e-06 10.1814476822772 -15.706282608207 5.36616625579633;-1.89e-06 10.181431112492 -15.7062368864293 5.36626471856739;-1.88e-06 10.181414542562 -15.7061911644285 5.36636318126219;-1.87e-06 10.1813979724873 -15.7061454422044 5.36646164388072;-1.86e-06 10.181381402268 -15.7060997197571 5.36656010642299;-1.85e-06 10.1813648319039 -15.7060539970866 5.36665856888898;-1.84e-06 10.1813482613952 -15.7060082741929 5.3667570312787;-1.83e-06 10.1813316907417 -15.7059625510759 5.36685549359214;-1.82e-06 10.1813151199436 -15.7059168277357 5.36695395582931;-1.81e-06 10.1812985490007 -15.7058711041724 5.36705241799021;-1.8e-06 10.1812819779132 -15.7058253803858 5.36715088007483;-1.79e-06 10.1812654066809 -15.7057796563759 5.36724934208316;-1.78e-06 10.181248835304 -15.7057339321429 5.36734780401522;-1.77e-06 10.1812322637823 -15.7056882076867 5.367446265871;-1.76e-06 10.181215692116 -15.7056424830072 5.36754472765049;-1.75e-06 10.1811991203049 -15.7055967581045 5.36764318935369;-1.74e-06 10.1811825483492 -15.7055510329787 5.36774165098062;-1.73e-06 10.1811659762488 -15.7055053076296 5.36784011253125;-1.72e-06 10.1811494040036 -15.7054595820573 5.36793857400559;-1.71e-06 10.1811328316138 -15.7054138562618 5.36803703540365;-1.7e-06 10.1811162590793 -15.705368130243 5.36813549672541;-1.69e-06 10.1810996864 -15.7053224040011 5.36823395797088;-1.68e-06 10.1810831135761 -15.705276677536 5.36833241914005;-1.67e-06 10.1810665406075 -15.7052309508476 5.36843088023293;-1.66e-06 10.1810499674942 -15.7051852239361 5.36852934124951;-1.65e-06 10.1810333942362 -15.7051394968013 5.36862780218979;-1.64e-06 10.1810168208335 -15.7050937694434 5.36872626305378;-1.63e-06 10.1810002472861 -15.7050480418622 5.36882472384146;-1.62e-06 10.180983673594 -15.7050023140578 5.36892318455283;-1.61e-06 10.1809670997572 -15.7049565860303 5.36902164518791;-1.6e-06 10.1809505257757 -15.7049108577795 5.36912010574667;-1.59e-06 10.1809339516495 -15.7048651293055 5.36921856622913;-1.58e-06 10.1809173773787 -15.7048194006083 5.36931702663529;-1.57e-06 10.1809008029631 -15.704773671688 5.36941548696513;-1.56e-06 10.1808842284028 -15.7047279425444 5.36951394721866;-1.55e-06 10.1808676536979 -15.7046822131776 5.36961240739588;-1.54e-06 10.1808510788483 -15.7046364835876 5.36971086749678;-1.53e-06 10.1808345038539 -15.7045907537745 5.36980932752137;-1.52e-06 10.1808179287149 -15.7045450237381 5.36990778746964;-1.51e-06 10.1808013534312 -15.7044992934785 5.37000624734159;-1.5e-06 ...(+9089 chars)]  x4=[-2.33e-06 -7.35211206581993 0 0;-2.32e-06 -7.352099778351 0 0;-2.31e-06 -7.35208749077758 0 0;-2.3e-06 -7.35207520309967 0 0;-2.29e-06 -7.35206291531727 0 0;-2.28e-06 -7.35205062743039 0 0;-2.27e-06 -7.35203833943901 0 0;-2.26e-06 -7.35202605134315 0 0;-2.25e-06 -7.35201376314279 0 0;-2.24e-06 -7.35200147483795 0 0;-2.23e-06 -7.35198918642862 0 0;-2.22e-06 -7.3519768979148 0 0;-2.21e-06 -7.3519646092965 0 0;-2.2e-06 -7.3519523205737 0 0;-2.19e-06 -7.35194003174642 0 0;-2.18e-06 -7.35192774281465 0 0;-2.17e-06 -7.3519154537784 0 0;-2.16e-06 -7.35190316463765 0 0;-2.15e-06 -7.35189087539242 0 0;-2.14e-06 -7.3518785860427 0 0;-2.13e-06 -7.3518662965885 0 0;-2.12e-06 -7.35185400702981 0 0;-2.11e-06 -7.35184171736663 0 0;-2.1e-06 -7.35182942759897 0 0;-2.09e-06 -7.35181713772682 0 0;-2.08e-06 -7.35180484775019 0 0;-2.07e-06 -7.35179255766907 0 0;-2.06e-06 -7.35178026748346 0 0;-2.05e-06 -7.35176797719337 0 0;-2.04e-06 -7.3517556867988 0 0;-2.03e-06 -7.35174339629974 0 0;-2.02e-06 -7.35173110569619 0 0;-2.01e-06 -7.35171881498816 0 0;-2e-06 -7.35170652417565 0 0;-1.99e-06 -7.35169423325865 0 0;-1.98e-06 -7.35168194223717 61.2880909242956 0;-1.97e-06 -7.35166965111121 61.2886083009315 0;-1.96e-06 -7.35165735988076 61.2891256766963 0;-1.95e-06 -7.35164506854582 61.28964305159 118.244738296023;-1.94e-06 -7.35163277710641 61.2901604256127 118.244496042452;-1.93e-06 -7.35162048556251 61.2906777987644 118.2442537872;-1.92e-06 -7.35160819391413 61.2911951710449 118.244011530268;-1.91e-06 -7.35159590216126 61.2917125424544 118.243769271656;-1.9e-06 -7.35158361030392 61.2922299129927 118.243527011363;-1.89e-06 -7.35157131834209 61.29274728266 118.243284749389;-1.88e-06 -7.35155902627578 61.2932646514562 118.243042485735;-1.87e-06 -7.35154673410498 61.2937820193812 118.242800220401;-1.86e-06 -7.35153444182971 61.2942993864351 118.242557953386;-1.85e-06 -7.35152214944995 61.2948167526179 118.24231568469;-1.84e-06 -7.35150985696571 61.2953341179296 118.242073414314;-1.83e-06 -7.35149756437699 61.2958514823701 118.241831142258;-1.82e-06 -7.35148527168379 61.2963688459395 118.241588868521;-1.81e-06 -7.3514729788861 61.2968862086377 118.241346593104;-1.8e-06 -7.35146068598394 61.2974035704647 118.241104316006;-1.79e-06 -7.3514483929773 61.2979209314206 118.240862037227;-1.78e-06 -7.35143609986617 61.2984382915053 118.240619756768;-1.77e-06 -7.35142380665057 61.2989556507188 118.240377474629;-1.76e-06 -7.35141151333048 61.2994730090611 118.240135190809;-1.75e-06 -7.35139921990592 61.2999903665322 118.239892905309;-1.74e-06 -7.35138692637687 61.3005077231321 118.239650618129;-1.73e-06 -7.35137463274335 61.3010250788608 118.239408329268;-1.72e-06 -7.35136233900534 61.3015424337182 118.239166038726;-1.71e-06 -7.35135004516286 61.3020597877044 118.238923746504;-1.7e-06 -7.3513377512159 61.3025771408194 118.238681452602;-1.69e-06 -7.35132545716446 61.3030944930631 118.238439157019;-1.68e-06 -7.35131316300854 61.3036118444356 118.238196859756;-1.67e-06 -7.35130086874814 61.3041291949369 118.237954560812;-1.66e-06 -7.35128857438326 61.3046465445668 118.237712260188;-1.65e-06 -7.35127627991391 61.3051638933255 118.237469957883;-1.64e-06 -7.35126398534008 61.3056812412129 118.237227653898;-1.63e-06 -7.35125169066177 61.306198588229 118.236985348233;-1.62e-06 -7.35123939587898 61.3067159343738 118.236743040887;-1.61e-06 -7.35122710099171 61.3072332796473 118.236500731861;-1.6e-06 -7.35121480599997 61.3077506240495 118.236258421154;-1.59e-06 -7.35120251090375 61.3082679675803 118.236016108767;-1.58e-06 -7.35119021570305 61.3087853102399 118.2357737947;-1.57e-06 -7.35117792039788 61.3093026520281 118.235531478952;-1.56e-06 -7.35116562498823 61.309819992945 118.235289161524;-1.55e-06 -7.3511533294741 61.3103373329905 118.235046842415;-1.54e-06 -7.3511410338555 61.3108546721646 118.234804521626;-1.53e-06 -7.35112873813242 61.3113720104674 118.234562199157;-1.52e-06 -7.35111644230486 61.3118893478988 118.234319875007;-1.51e-06 -7.35110414637283 61.3124066844589 ...(+9131 chars)]  x5=2.32157794725242e-06  V1=ones(3,3)  V2=ones(3,3)  I1=ones(3,3)  I2=ones(3,3)  nHarmo=1  Measurements=None  Decoupling=off  VsMag0=0  VrMag0=0  VsAngle0=0  VrAngle0=0  IsMag0=0  IrMag0=0  IsAngle0=0  IrAngle0=0
      settings: LibrarySourceBlock=sps_lib/Power Grid Elements/Distributed Parameters Line
  806 Yg PQ : spsThreePhaseSeriesRLCLoadLib/Three-Phase Series RLC Load  LConnTagsString=A|B|C  Configuration=Y (grounded)  NominalVoltage=[  Vabc=V_802_806*Vnom  Vabcp=[1e3 1e3 1e3]  NominalFrequency=Frequency  UnbalancedPower=on  ActivePower=10e3  InductivePower=100  CapacitivePower=100  Pabc=Pa_802_806/2  QLabc=Pp_802_806/2  QCabc=Pn_802_806  Pabcp=[10e3 9e3 11e3]  QLabcp=[100 90 110]  QCabcp=[100 90 110]  Measurements=None  LoadType=constant PQ
      settings: AttributesFormatString=|
      | P= %<Pabc> W
      | Ql=%<QLabc> var
      | Qc=%<QCabc> var